simulink model "testDirect"  (Simulink 7.6)
settings: Solver=ode45  StartTime=0.0  StopTime=1400.0  RelTol=1e-3
libraries: xbsIndex_r4 x2187, casper_library x117, monroe_library x15, xps_library x1
(part 2 of 20)

== system testDirect/complex_scope7 ==
  In1 : Inport
  Gateway Out15 : xbsIndex_r4/Gateway Out  hdl_port=on  timing_constraint=None  locs_specified=off  LOCs={}  block_type=gatewayout
      settings: infoedit="Gateway out block.  Converts Xilinx fixed point inputs into ouputs of type Simulink integer, double, or fixed point.<br><br>Hardware notes:  In hardware these blocks become top level output ports or are discarded, depending on how they are configured."  block_version=11.4
  Gateway Out16 : xbsIndex_r4/Gateway Out  hdl_port=on  timing_constraint=None  locs_specified=off  LOCs={}  block_type=gatewayout
      settings: infoedit="Gateway out block.  Converts Xilinx fixed point inputs into ouputs of type Simulink integer, double, or fixed point.<br><br>Hardware notes:  In hardware these blocks become top level output ports or are discarded, depending on how they are configured."  block_version=11.4
  Reinterpret : xbsIndex_r4/Reinterpret  force_arith_type=on  arith_type="Signed  (2's comp)"  force_bin_pt=on  bin_pt=17  block_type=reinterpret
      settings: infoedit="Changes signal type without altering the binary representation.   You can changed the signal between signed and unsigned, and relocate the binary point.<br><br>Hardware notes: In hardware this block costs nothing.<br><br>Example:  Suppose the input is 6 bits wide, signed, with 2 fractional bits, and the output is forced to unsigned with 0 fractional bits.  Then an input of -2.0 (1110.00 in binary 2's complement) becomes an output of 56 (111000 in binary)."
  Reinterpret1 : xbsIndex_r4/Reinterpret  force_arith_type=on  arith_type="Signed  (2's comp)"  force_bin_pt=on  bin_pt=17  block_type=reinterpret
      settings: infoedit="Changes signal type without altering the binary representation.   You can changed the signal between signed and unsigned, and relocate the binary point.<br><br>Hardware notes: In hardware this block costs nothing.<br><br>Example:  Suppose the input is 6 bits wide, signed, with 2 fractional bits, and the output is forced to unsigned with 0 fractional bits.  Then an input of -2.0 (1110.00 in binary 2's complement) becomes an output of 56 (111000 in binary)."
  c_to_ri : casper_library/Misc/c_to_ri  SystemSampleTime=-1  n_bits=18  bin_pt=17
      settings: AttributesFormatString=18_17 r/i
  Real : Outport
  Imag : Outport  Port=2
  wires:
  Gateway Out16/1 -> Imag/1
  In1/1 -> c_to_ri/1
  Gateway Out15/1 -> Real/1
  Reinterpret1/1 -> Gateway Out16/1
  Reinterpret/1 -> Gateway Out15/1
  c_to_ri/2 -> Reinterpret1/1
  c_to_ri/1 -> Reinterpret/1

== system testDirect/complex_scope8 ==
  In1 : Inport
  Gateway Out15 : xbsIndex_r4/Gateway Out  hdl_port=on  timing_constraint=None  locs_specified=off  LOCs={}  block_type=gatewayout
      settings: infoedit="Gateway out block.  Converts Xilinx fixed point inputs into ouputs of type Simulink integer, double, or fixed point.<br><br>Hardware notes:  In hardware these blocks become top level output ports or are discarded, depending on how they are configured."  block_version=11.4
  Gateway Out16 : xbsIndex_r4/Gateway Out  hdl_port=on  timing_constraint=None  locs_specified=off  LOCs={}  block_type=gatewayout
      settings: infoedit="Gateway out block.  Converts Xilinx fixed point inputs into ouputs of type Simulink integer, double, or fixed point.<br><br>Hardware notes:  In hardware these blocks become top level output ports or are discarded, depending on how they are configured."  block_version=11.4
  Reinterpret : xbsIndex_r4/Reinterpret  force_arith_type=on  arith_type="Signed  (2's comp)"  force_bin_pt=on  bin_pt=17  block_type=reinterpret
      settings: infoedit="Changes signal type without altering the binary representation.   You can changed the signal between signed and unsigned, and relocate the binary point.<br><br>Hardware notes: In hardware this block costs nothing.<br><br>Example:  Suppose the input is 6 bits wide, signed, with 2 fractional bits, and the output is forced to unsigned with 0 fractional bits.  Then an input of -2.0 (1110.00 in binary 2's complement) becomes an output of 56 (111000 in binary)."
  Reinterpret1 : xbsIndex_r4/Reinterpret  force_arith_type=on  arith_type="Signed  (2's comp)"  force_bin_pt=on  bin_pt=17  block_type=reinterpret
      settings: infoedit="Changes signal type without altering the binary representation.   You can changed the signal between signed and unsigned, and relocate the binary point.<br><br>Hardware notes: In hardware this block costs nothing.<br><br>Example:  Suppose the input is 6 bits wide, signed, with 2 fractional bits, and the output is forced to unsigned with 0 fractional bits.  Then an input of -2.0 (1110.00 in binary 2's complement) becomes an output of 56 (111000 in binary)."
  c_to_ri : casper_library/Misc/c_to_ri  SystemSampleTime=-1  n_bits=18  bin_pt=17
      settings: AttributesFormatString=18_17 r/i
  Real : Outport
  Imag : Outport  Port=2
  wires:
  c_to_ri/1 -> Reinterpret/1
  c_to_ri/2 -> Reinterpret1/1
  Reinterpret/1 -> Gateway Out15/1
  Reinterpret1/1 -> Gateway Out16/1
  Gateway Out15/1 -> Real/1
  In1/1 -> c_to_ri/1
  Gateway Out16/1 -> Imag/1

== system testDirect/complex_scope9 ==
  In1 : Inport
  Gateway Out15 : xbsIndex_r4/Gateway Out  hdl_port=on  timing_constraint=None  locs_specified=off  LOCs={}  block_type=gatewayout
      settings: infoedit="Gateway out block.  Converts Xilinx fixed point inputs into ouputs of type Simulink integer, double, or fixed point.<br><br>Hardware notes:  In hardware these blocks become top level output ports or are discarded, depending on how they are configured."  block_version=11.4
  Gateway Out16 : xbsIndex_r4/Gateway Out  hdl_port=on  timing_constraint=None  locs_specified=off  LOCs={}  block_type=gatewayout
      settings: infoedit="Gateway out block.  Converts Xilinx fixed point inputs into ouputs of type Simulink integer, double, or fixed point.<br><br>Hardware notes:  In hardware these blocks become top level output ports or are discarded, depending on how they are configured."  block_version=11.4
  Reinterpret : xbsIndex_r4/Reinterpret  force_arith_type=on  arith_type="Signed  (2's comp)"  force_bin_pt=on  bin_pt=17  block_type=reinterpret
      settings: infoedit="Changes signal type without altering the binary representation.   You can changed the signal between signed and unsigned, and relocate the binary point.<br><br>Hardware notes: In hardware this block costs nothing.<br><br>Example:  Suppose the input is 6 bits wide, signed, with 2 fractional bits, and the output is forced to unsigned with 0 fractional bits.  Then an input of -2.0 (1110.00 in binary 2's complement) becomes an output of 56 (111000 in binary)."
  Reinterpret1 : xbsIndex_r4/Reinterpret  force_arith_type=on  arith_type="Signed  (2's comp)"  force_bin_pt=on  bin_pt=17  block_type=reinterpret
      settings: infoedit="Changes signal type without altering the binary representation.   You can changed the signal between signed and unsigned, and relocate the binary point.<br><br>Hardware notes: In hardware this block costs nothing.<br><br>Example:  Suppose the input is 6 bits wide, signed, with 2 fractional bits, and the output is forced to unsigned with 0 fractional bits.  Then an input of -2.0 (1110.00 in binary 2's complement) becomes an output of 56 (111000 in binary)."
  c_to_ri : casper_library/Misc/c_to_ri  SystemSampleTime=-1  n_bits=18  bin_pt=17
      settings: AttributesFormatString=18_17 r/i
  Real : Outport
  Imag : Outport  Port=2
  wires:
  Gateway Out16/1 -> Imag/1
  In1/1 -> c_to_ri/1
  Gateway Out15/1 -> Real/1
  Reinterpret1/1 -> Gateway Out16/1
  Reinterpret/1 -> Gateway Out15/1
  c_to_ri/2 -> Reinterpret1/1
  c_to_ri/1 -> Reinterpret/1

== system testDirect/fft_direct ==
  sync : Inport
  in0 : Inport  Port=2
  in1 : Inport  Port=3
  in2 : Inport  Port=4
  in3 : Inport  Port=5
  in4 : Inport  Port=6
  in5 : Inport  Port=7
  in6 : Inport  Port=8
  in7 : Inport  Port=9
  bulk_delay_0_1 : Subsystem
  bulk_delay_1_1 : Subsystem
  bulk_delay_2_1 : Subsystem
  bulk_delay_3_1 : Subsystem
  direct_coeff_gen : Subsystem
  stage_1 : Subsystem
  stage_2 : Subsystem
  stage_3 : Subsystem
  sync_out : Outport
  out0 : Outport  Port=2
  out1 : Outport  Port=3
  out2 : Outport  Port=4
  out3 : Outport  Port=5
  out4 : Outport  Port=6
  out5 : Outport  Port=7
  out6 : Outport  Port=8
  out7 : Outport  Port=9
  wires:
  bulk_delay_3_1/8(out8) -> out7/1
  bulk_delay_3_1/7(out7) -> out6/1
  bulk_delay_3_1/6(out6) -> out5/1
  bulk_delay_3_1/5(out5) -> out4/1
  bulk_delay_3_1/4(out4) -> out3/1
  bulk_delay_3_1/3(out3) -> out2/1
  bulk_delay_3_1/2(out2) -> out1/1
  bulk_delay_3_1/1(out1) -> out0/1
  stage_3/8(AmBW_3) -> bulk_delay_3_1/8(in8)
  stage_3/4(AmBW_1) -> bulk_delay_3_1/7(in7)
  stage_3/6(AmBW_2) -> bulk_delay_3_1/6(in6)
  stage_3/2(AmBW_0) -> bulk_delay_3_1/5(in5)
  stage_3/7(ApBW_3) -> bulk_delay_3_1/4(in4)
  stage_3/3(ApBW_1) -> bulk_delay_3_1/3(in3)
  stage_3/5(ApBW_2) -> bulk_delay_3_1/2(in2)
  stage_3/1(ApBW_0) -> bulk_delay_3_1/1(in1)
  direct_coeff_gen/3(coeff_stg3) -> stage_3/9(coeff_arr)
  bulk_delay_2_1/8(out8) -> stage_3/8(in3_B)
  bulk_delay_2_1/7(out7) -> stage_3/7(in3_A)
  bulk_delay_2_1/6(out6) -> stage_3/6(in2_B)
  bulk_delay_2_1/5(out5) -> stage_3/5(in2_A)
  bulk_delay_2_1/4(out4) -> stage_3/4(in1_B)
  bulk_delay_2_1/3(out3) -> stage_3/3(in1_A)
  bulk_delay_2_1/2(out2) -> stage_3/2(in0_B)
  bulk_delay_2_1/1(out1) -> stage_3/1(in0_A)
  stage_2/8(AmBW_3) -> bulk_delay_2_1/8(in8)
  stage_2/6(AmBW_2) -> bulk_delay_2_1/7(in7)
  stage_2/7(ApBW_3) -> bulk_delay_2_1/6(in6)
  stage_2/5(ApBW_2) -> bulk_delay_2_1/5(in5)
  stage_2/4(AmBW_1) -> bulk_delay_2_1/4(in4)
  stage_2/2(AmBW_0) -> bulk_delay_2_1/3(in3)
  stage_2/3(ApBW_1) -> bulk_delay_2_1/2(in2)
  stage_2/1(ApBW_0) -> bulk_delay_2_1/1(in1)
  direct_coeff_gen/2(coeff_stg2) -> stage_2/9(coeff_arr)
  bulk_delay_1_1/8(out8) -> stage_2/8(in3_B)
  bulk_delay_1_1/7(out7) -> stage_2/7(in3_A)
  bulk_delay_1_1/6(out6) -> stage_2/6(in2_B)
  bulk_delay_1_1/5(out5) -> stage_2/5(in2_A)
  bulk_delay_1_1/4(out4) -> stage_2/4(in1_B)
  bulk_delay_1_1/3(out3) -> stage_2/3(in1_A)
  bulk_delay_1_1/2(out2) -> stage_2/2(in0_B)
  bulk_delay_1_1/1(out1) -> stage_2/1(in0_A)
  stage_1/8(AmBW_3) -> bulk_delay_1_1/8(in8)
  stage_1/4(AmBW_1) -> bulk_delay_1_1/7(in7)
  stage_1/6(AmBW_2) -> bulk_delay_1_1/6(in6)
  stage_1/2(AmBW_0) -> bulk_delay_1_1/5(in5)
  stage_1/7(ApBW_3) -> bulk_delay_1_1/4(in4)
  stage_1/3(ApBW_1) -> bulk_delay_1_1/3(in3)
  stage_1/5(ApBW_2) -> bulk_delay_1_1/2(in2)
  stage_1/1(ApBW_0) -> bulk_delay_1_1/1(in1)
  direct_coeff_gen/1(coeff_stg1) -> stage_1/9(coeff_arr)
  bulk_delay_0_1/8(out8) -> stage_1/8(in3_B)
  bulk_delay_0_1/7(out7) -> stage_1/7(in3_A)
  bulk_delay_0_1/6(out6) -> stage_1/6(in2_B)
  bulk_delay_0_1/5(out5) -> stage_1/5(in2_A)
  bulk_delay_0_1/4(out4) -> stage_1/4(in1_B)
  bulk_delay_0_1/3(out3) -> stage_1/3(in1_A)
  bulk_delay_0_1/2(out2) -> stage_1/2(in0_B)
  bulk_delay_0_1/1(out1) -> stage_1/1(in0_A)
  direct_coeff_gen/4(sync_out) -> sync_out/1
  sync/1 -> direct_coeff_gen/1(sync)
  in7/1 -> bulk_delay_0_1/8(in8)
  in3/1 -> bulk_delay_0_1/7(in7)
  in6/1 -> bulk_delay_0_1/6(in6)
  in2/1 -> bulk_delay_0_1/5(in5)
  in5/1 -> bulk_delay_0_1/4(in4)
  in1/1 -> bulk_delay_0_1/3(in3)
  in4/1 -> bulk_delay_0_1/2(in2)
  in0/1 -> bulk_delay_0_1/1(in1)
